simulink model "wlan_phy_rx_pmd"  (Simulink 7.8)
settings: Solver=VariableStepDiscrete  SolverMode=SingleTasking  StartTime=0.0  StopTime=rx_sim_time  RelTol=1e-3
PostLoadFcn:
  | wlan_phy_rx_init
InitFcn:
  | wlan_phy_rx_init
StartFcn:
  | wlan_phy_rx_init
values from neighbouring files (symbols this model uses):
  barker_seq20 = 0.95 * circshift(barker_seq20, [0 4]) ./ max(abs(barker_seq20))    [wlan_phy_rx_init.m]
  bit_scrambler_lfsr_bytes = bi2de(reshape(repmat(scr, 1, 8), 8, 127)', 'left-msb')    [wlan_phy_rx_init.m]
  deinterleave_ROM = [deinterleave_ROM interleave_64QAM zeros(1, 512-length(interleave_64QAM))]    [wlan_phy_rx_init.m]
  longCorr_coef_i = [3*real(longCorr_coef)]    [wlan_phy_rx_init.m]
  longCorr_coef_q = [3*imag(longCorr_coef)]    [wlan_phy_rx_init.m]
  MAX_CP_LEN = 32    [wlan_phy_rx_init.m]
  MAX_NCBPS = NCBPS_64QAM    (= 288)    [wlan_phy_rx_init.m]
  MAX_NUM_BYTES = 4096    [wlan_phy_rx_init.m]
  MAX_NUM_SC = 64    [wlan_phy_rx_init.m]
  MAX_NUM_SYMS = 600    [wlan_phy_rx_init.m]
  NCBPS_16QAM = 192    [wlan_phy_rx_init.m]
  NCBPS_64QAM = 288    [wlan_phy_rx_init.m]
  NCBPS_BPSK = 48    [wlan_phy_rx_init.m]
  NCBPS_QPSK = 96    [wlan_phy_rx_init.m]
  NDBPS_16QAM12 = 96    [wlan_phy_rx_init.m]
  NDBPS_16QAM34 = 144    [wlan_phy_rx_init.m]
  NDBPS_64QAM23 = 192    [wlan_phy_rx_init.m]
  NDBPS_64QAM34 = 216    [wlan_phy_rx_init.m]
  NDBPS_BPSK12 = 24    [wlan_phy_rx_init.m]
  NDBPS_BPSK34 = 36    [wlan_phy_rx_init.m]
  NDBPS_QPSK12 = 48    [wlan_phy_rx_init.m]
  NDBPS_QPSK34 = 72    [wlan_phy_rx_init.m]
  PHY_MIN_PKT_LEN = 14    [wlan_phy_rx_init.m]
  preFFT_sampBuff_numSamps = 4*MAX_NUM_SC    (= 256)    [wlan_phy_rx_init.m]
  rx_sim_sig_samp_time = 8    [wlan_phy_rx_init.m]
  scr_ind_rev = zeros(1,128)    [wlan_phy_rx_init.m]
undefined symbols (not defined in the file or in its folder): gcbh, xlBlockLoadCallback, xlGetNormalizedPeriod, xlipgetpartsetting
libraries: xbsIndex_r4 x3524, simulink x1, xbsEDKLib_r4 x1
(part 11 of 21)

== system wlan_phy_rx_pmd/Sync & Antenna Sel/LTS Correlation/Correlator ==
  note: The architecture for this correlator is based on one developed by Dr. Chris Dick (Cheif DSP Scientist at Xilinx).
      | The version here has been modified in a few ways:
      | -The inner correlators run at 8x the input sample rate (vs 5 in the original)
      | -The "multiplications" are 3-bit by 1-bit (vs 1x1 in the original), which improves
      | correlation performance with interference or overlapping preambles
  Rx I : Inport
  Rx Q : Inport  Port=2
  rx_IQ_valid : Inport  Port=3
  C1 : Subsystem  mask{N=8, h=longCorr_coef_i, Ts_In=8}
      mask prompts: N "Coefficients/correlator"; h "Coefficients"; Ts_In "Input sample period"
  C2 : Subsystem  mask{N=8, h=longCorr_coef_i, Ts_In=8}
      mask prompts: N "Coefficients/correlator"; h "Coefficients"; Ts_In "Input sample period"
  C3 : Subsystem  mask{N=8, h=longCorr_coef_q, Ts_In=8}
      mask prompts: N "Coefficients/correlator"; h "Coefficients"; Ts_In "Input sample period"
  C4 : Subsystem  mask{N=8, h=longCorr_coef_q, Ts_In=8}
      mask prompts: N "Coefficients/correlator"; h "Coefficients"; Ts_In "Input sample period"
  Combine : Subsystem
  Delay1 : xbsIndex_r4/Delay  rst=off  infoeditControl=Selection of Reset will increase slice count due to use of real FFs and instead of SRLs  en=on  latency=1  dbl_ovrd=off  reg_retiming=off  block_type=delay
  Delay2 : xbsIndex_r4/Delay  rst=off  infoeditControl=Selection of Reset will increase slice count due to use of real FFs and instead of SRLs  en=on  latency=1  dbl_ovrd=off  reg_retiming=off  block_type=delay
  Delay3 : xbsIndex_r4/Delay  rst=off  infoeditControl=Selection of Reset will increase slice count due to use of real FFs and instead of SRLs  en=off  latency=1  dbl_ovrd=off  reg_retiming=off  block_type=delay
  Delay4 : xbsIndex_r4/Delay  rst=off  infoeditControl=Selection of Reset will increase slice count due to use of real FFs and instead of SRLs  en=off  latency=1  dbl_ovrd=off  reg_retiming=off  block_type=delay
  FSM : Subsystem  mask{N=8}
      mask prompts: N "Coefficients/correlator"
  Goto Tag Visibility : GotoTagVisibility  GotoTag=ltsCorr_dAddr
  Goto Tag Visibility1 : GotoTagVisibility  GotoTag=ltsCorr_cAddr
  Goto Tag Visibility2 : GotoTagVisibility  GotoTag=ltsCorr_ld
  R : Outport
  tag scope: CS_LTS_Corr=global, ltsCorr_cAddr=scoped, ltsCorr_dAddr=scoped, ltsCorr_ld=scoped
  wires:
  rx_IQ_valid/1 -> Delay1/2, Delay2/2, Delay4/1, Delay3/1
  FSM/3(load) -> [ltsCorr_ld]
  FSM/2(Coef Addr) -> [ltsCorr_cAddr]
  FSM/1(Data Addr) -> [ltsCorr_dAddr]
  C3/1(y) -> Combine/2(Im*Im)
  Delay2/1 -> C2/1(x), C3/1(x)
  Delay1/1 -> C1/1(x), C4/1(x)
  C1/1(y) -> Combine/1(Re*Re)
  C2/1(y) -> Combine/4(Im*Re)
  C4/1(y) -> Combine/3(Re*Im)
  Combine/1(R) -> R/1, [CS_LTS_Corr]
  Rx I/1 -> Delay1/1
  Rx Q/1 -> Delay2/1
  Delay3/1 -> FSM/1(IQ_Valid)
  Delay4/1 -> C1/2(x_valid), C3/2(x_valid), C2/2(x_valid), C4/2(x_valid)

== system wlan_phy_rx_pmd/Sync & Antenna Sel/LTS Correlation/LTS Corr Timeout ==
  Pkt Det : Inport
  IQ Valid : Inport  Port=2
  LTS 1 : Inport  Port=3
  LTS 2 : Inport  Port=4
  Counter : xbsIndex_r4/Counter  cnt_type=Free Running  cnt_to=Inf  operation=Up  start_count=0  cnt_by_val=1  arith_type=Unsigned  n_bits=9  bin_pt=0  load_pin=off  rst=on  en=on  explicit_period=off  period=1  dbl_ovrd=off  use_behavioral_HDL=off  implementation=Fabric  block_type=counter
  Display : Display
  Gateway Out{1..9} (9 alike) : xbsIndex_r4/Gateway Out  inherit_from_input=off  hdl_port=off  timing_constraint=None  locs_specified=off  LOCs={}  UseAsDAC=off  DACChannel='1'  block_type=gatewayout
  Inverter : xbsIndex_r4/Inverter  en=off  latency=0  dbl_ovrd=off  block_type=inv
  LTS Timeout : Scope  NumInputPorts=10
      settings: TimeRange=18000  YMin=0~0~0~0~0~0~0~0~-1~-1  YMax=1~1~1~1~1~600~1~1~1~1  SaveName=ScopeData17
  Logical : xbsIndex_r4/Logical  logical_function=AND  inputs=2  en=off  latency=0  precision=Full  arith_type=Unsigned  n_bits=16  bin_pt=0  align_bp=on  dbl_ovrd=off  block_type=logical
  Logical1 : xbsIndex_r4/Logical  logical_function=NAND  inputs=2  en=off  latency=0  precision=Full  arith_type=Unsigned  n_bits=16  bin_pt=0  align_bp=on  dbl_ovrd=off  block_type=logical
  Logical2 : xbsIndex_r4/Logical  logical_function=AND  inputs=2  en=off  latency=0  precision=Full  arith_type=Unsigned  n_bits=16  bin_pt=0  align_bp=on  dbl_ovrd=off  block_type=logical
  Relational : xbsIndex_r4/Relational  mode=a>b  en=off  latency=0  dbl_ovrd=off  block_type=relational
  S-R Latch : Subsystem
  S-R Latch1 : Subsystem
  done14 : xbsIndex_r4/Gateway Out  inherit_from_input=off  hdl_port=off  timing_constraint=None  locs_specified=off  LOCs={}  UseAsDAC=off  DACChannel='1'  block_type=gatewayout
      settings: infoedit="Gateway out block.  Converts Xilinx fixed point inputs into ouputs of type Simulink integer, double, or fixed point.<P><P>Hardware notes:  In hardware these blocks become top level output ports or are discarded, depending on how they are configured."  xl_area=[0 0 0 0 1 0 0]  block_version=VER_STRING_GOES_HERE
  Timeout : Outport
  wires:
  Logical/1 -> Counter/2
  Pkt Det/1 -> Logical/1, Inverter/1, Gateway Out5/1
  IQ Valid/1 -> Logical/2
  Inverter/1 -> Counter/1, S-R Latch/2(R), S-R Latch1/2(R)
  LTS 1/1 -> S-R Latch/1(S), Gateway Out4/1
  LTS 2/1 -> S-R Latch1/1(S), Gateway Out1/1
  S-R Latch/1(Q) -> Logical1/1, Gateway Out2/1
  S-R Latch1/1(Q) -> Logical1/2, Gateway Out3/1
  Logical1/1 -> Logical2/1, Gateway Out8/1
  Relational/1 -> Logical2/2, Gateway Out9/1
  Logical2/1 -> Timeout/1, Gateway Out7/1
  Counter/1 -> Relational/1, Gateway Out6/1
  [regRx_PktDet_Timout] -> Relational/2, done14/1
  done14/1 -> Display/1
  Gateway Out4/1 -> LTS Timeout/1
  Gateway Out2/1 -> LTS Timeout/2
  Gateway Out1/1 -> LTS Timeout/3
  Gateway Out3/1 -> LTS Timeout/4
  Gateway Out5/1 -> LTS Timeout/5
  Gateway Out6/1 -> LTS Timeout/6
  Gateway Out7/1 -> LTS Timeout/10
  Gateway Out8/1 -> LTS Timeout/7
  Gateway Out9/1 -> LTS Timeout/8

== system wlan_phy_rx_pmd/Sync & Antenna Sel/LTS Correlation/Posedge3 ==
  note: Rising Edge Detection
  D : Inport
  Delay : xbsIndex_r4/Delay  rst=off  infoeditControl=Selection of Reset will increase slice count due to use of real FFs and instead of SRLs  en=off  latency=1  dbl_ovrd=off  reg_retiming=off  block_type=delay
      settings: infoedit=Hardware notes: A delay line is a chain, each link of which is an SRL16 followed by a flip-flop. If register retiming is enabled, the delay line is a chain of flip-flops.  xl_area=[1 1 0 0 0 0 0]  block_version=VER_STRING_GOES_HERE
  Inverter : xbsIndex_r4/Inverter  en=off  latency=0  dbl_ovrd=off  block_type=inv
      settings: xl_area=[0 0 0 0 0 0 0]  block_version=VER_STRING_GOES_HERE
  Logical1 : xbsIndex_r4/Logical  logical_function=AND  inputs=2  en=off  latency=0  precision=Full  arith_type=Unsigned  n_bits=8  bin_pt=2  align_bp=on  dbl_ovrd=off  block_type=logical
      settings: xl_area=[1 0 0 1 0 0 0]  block_version=VER_STRING_GOES_HERE
  Q : Outport
  wires:
  D/1 -> Logical1/2, Delay/1
  Logical1/1 -> Q/1
  Inverter/1 -> Logical1/1
  Delay/1 -> Inverter/1

== system wlan_phy_rx_pmd/Sync & Antenna Sel/LTS Correlation/S-R Latch ==
  S : Inport
  R : Inport  Port=2
  Constant1 : xbsIndex_r4/Constant  const=1  gui_display_data_type=Fixed-point  arith_type=Boolean  n_bits=1  bin_pt=0  preci_type=Single  exp_width=8  frac_width=24  explicit_period=off  period=1  equ=P=C  opselect=C  inp2=PCIN>>17  opr=+  inp1=P  carry=CIN  dbl_ovrd=off  block_type=constant
      settings: block_version=VER_STRING_GOES_HERE
  Convert1 : xbsIndex_r4/Convert  gui_display_data_type=Boolean  arith_type=Boolean  n_bits=1  bin_pt=0  float_type=Single  exp_bits=8  fraction_bits=24  quantization=Truncate  overflow=Wrap  en=off  latency=0  dbl_ovrd=off  pipeline=off  block_type=convert
  Convert2 : xbsIndex_r4/Convert  gui_display_data_type=Boolean  arith_type=Boolean  n_bits=1  bin_pt=0  float_type=Single  exp_bits=8  fraction_bits=24  quantization=Truncate  overflow=Wrap  en=off  latency=0  dbl_ovrd=off  pipeline=off  block_type=convert
  Register2 : xbsIndex_r4/Register  init=0  rst=on  en=on  dbl_ovrd=off  block_type=register
      settings: xl_area=[1 1 0 0 0 0 0]  block_version=VER_STRING_GOES_HERE
  Q : Outport
  wires:
  Convert2/1 -> Register2/3
  Convert1/1 -> Register2/2
  S/1 -> Convert2/1
  Register2/1 -> Q/1
  R/1 -> Convert1/1
  Constant1/1 -> Register2/1

== system wlan_phy_rx_pmd/Sync & Antenna Sel/LTS Correlation/S-R Latch1 ==
  S : Inport
  R : Inport  Port=2
  Constant1 : xbsIndex_r4/Constant  const=1  gui_display_data_type=Fixed-point  arith_type=Boolean  n_bits=1  bin_pt=0  preci_type=Single  exp_width=8  frac_width=24  explicit_period=off  period=1  equ=P=C  opselect=C  inp2=PCIN>>17  opr=+  inp1=P  carry=CIN  dbl_ovrd=off  block_type=constant
      settings: block_version=VER_STRING_GOES_HERE
  Convert1 : xbsIndex_r4/Convert  gui_display_data_type=Boolean  arith_type=Boolean  n_bits=1  bin_pt=0  float_type=Single  exp_bits=8  fraction_bits=24  quantization=Truncate  overflow=Wrap  en=off  latency=0  dbl_ovrd=off  pipeline=off  block_type=convert
  Convert2 : xbsIndex_r4/Convert  gui_display_data_type=Boolean  arith_type=Boolean  n_bits=1  bin_pt=0  float_type=Single  exp_bits=8  fraction_bits=24  quantization=Truncate  overflow=Wrap  en=off  latency=0  dbl_ovrd=off  pipeline=off  block_type=convert
  Register2 : xbsIndex_r4/Register  init=0  rst=on  en=on  dbl_ovrd=off  block_type=register
      settings: xl_area=[1 1 0 0 0 0 0]  block_version=VER_STRING_GOES_HERE
  Q : Outport
  wires:
  Constant1/1 -> Register2/1
  R/1 -> Convert1/1
  Register2/1 -> Q/1
  S/1 -> Convert2/1
  Convert1/1 -> Register2/2
  Convert2/1 -> Register2/3

== system wlan_phy_rx_pmd/Sync & Antenna Sel/LTS Correlation/Threshold Select ==
  Pkt Det : Inport
  RSSI : Inport  Port=2
  Mux : xbsIndex_r4/Mux  inputs=2  en=off  latency=0  precision=Full  arith_type=Unsigned  n_bits=16  bin_pt=14  quantization=Truncate  overflow=Wrap  dbl_ovrd=off  block_type=mux
  Register1 : xbsIndex_r4/Register  init=0  rst=off  en=on  dbl_ovrd=off  block_type=register
  Relational1 : xbsIndex_r4/Relational  mode=a>b  en=off  latency=0  dbl_ovrd=off  block_type=relational
      settings: xl_area=[10 0 0 19 0 0 0]  block_version=VER_STRING_GOES_HERE
  posedge : Subsystem
  Corr Thresh : Outport
  wires:
  Relational1/1 -> Mux/1
  RSSI/1 -> Register1/1
  posedge/1(Q) -> Register1/2
  Register1/1 -> Relational1/1
  [regRx_LongCorr_RSSI_Thresh] -> Relational1/2
  [regRx_LongCorr_Thresh_highSNR] -> Mux/3
  [regRx_LongCorr_Thresh_lowSNR] -> Mux/2
  Pkt Det/1 -> posedge/1(D)
  Mux/1 -> Corr Thresh/1

== system wlan_phy_rx_pmd/Sync & Antenna Sel/PHY CCA/PHY CCA Logic ==
  note: Two modes for physical carrier sensing:
      | Mode 0: Any enabled antenna can assert BUSY if its RSSI exceeds the threshold
      | Mode 1: BUSY only if *all* enabled antennas exceed the threshold
  note: This 4-in OR deasserts BUSY
      | if all antennas are disabled.
  Det A : Inport
  Det B : Inport  Port=2
  Det C : Inport  Port=3
  Det D : Inport  Port=4
  Inverter{,1..3} (4 alike) : xbsIndex_r4/Inverter  en=off  latency=0  dbl_ovrd=off  block_type=inv
  Logical{,2..4} (4 alike) : xbsIndex_r4/Logical  logical_function=AND  inputs=2  en=off  latency=0  precision=Full  arith_type=Unsigned  n_bits=16  bin_pt=0  align_bp=on  dbl_ovrd=off  block_type=logical
  Logical1 : xbsIndex_r4/Logical  logical_function=OR  inputs=4  en=off  latency=0  precision=Full  arith_type=Unsigned  n_bits=16  bin_pt=0  align_bp=on  dbl_ovrd=off  block_type=logical
  Logical10 : xbsIndex_r4/Logical  logical_function=OR  inputs=4  en=off  latency=0  precision=Full  arith_type=Unsigned  n_bits=16  bin_pt=0  align_bp=on  dbl_ovrd=off  block_type=logical
  Logical5 : xbsIndex_r4/Logical  logical_function=AND  inputs=5  en=off  latency=0  precision=Full  arith_type=Unsigned  n_bits=16  bin_pt=0  align_bp=on  dbl_ovrd=off  block_type=logical
  Logical{6..9} (4 alike) : xbsIndex_r4/Logical  logical_function=OR  inputs=2  en=off  latency=0  precision=Full  arith_type=Unsigned  n_bits=16  bin_pt=0  align_bp=on  dbl_ovrd=off  block_type=logical
  Mux : xbsIndex_r4/Mux  inputs=2  en=off  latency=0  precision=Full  arith_type=Unsigned  n_bits=16  bin_pt=14  quantization=Truncate  overflow=Wrap  dbl_ovrd=off  block_type=mux
  BUSY : Outport
  wires:
  [regRx_pktDetEn_RFA] -> Logical/1
  Logical/1 -> Logical1/1, Logical6/1
  Logical2/1 -> Logical1/2, Logical7/1
  Logical1/1 -> Mux/2
  Det A/1 -> Logical/2
  Det B/1 -> Logical2/2
  [regRx_pktDetEn_RFB] -> Logical2/1
  Det C/1 -> Logical3/2
  Det D/1 -> Logical4/2
  [regRx_pktDetEn_RFC] -> Logical3/1
  [regRx_pktDetEn_RFD] -> Logical4/1
  Logical3/1 -> Logical1/3, Logical8/1
  Logical4/1 -> Logical1/4, Logical9/1
  Inverter/1 -> Logical6/2
  [regRx_pktDetEn_RFA] -> Inverter/1, Logical10/1
  Logical6/1 -> Logical5/1
  Logical7/1 -> Logical5/2
  [regRx_pktDetEn_RFB] -> Inverter1/1, Logical10/2
  Inverter1/1 -> Logical7/2
  Logical8/1 -> Logical5/3
  [regRx_pktDetEn_RFC] -> Inverter2/1, Logical10/3
  Inverter2/1 -> Logical8/2
  [regRx_pktDetEn_RFD] -> Inverter3/1, Logical10/4
  Inverter3/1 -> Logical9/2
  Logical9/1 -> Logical5/4
  Logical10/1 -> Logical5/5
  Logical5/1 -> Mux/3
  Mux/1 -> BUSY/1
  [regRx_phyCCA_Mode_Sel] -> Mux/1

== system wlan_phy_rx_pmd/Sync & Antenna Sel/PHY CCA/Rate*Length BUSY ==
  note: CCA_BUSY for any Rx pkt with valid SIGNAL field
      | Rx Active drops after last data-bearing sample plus
      | programmable extension. This block doesn't care about
      | actual decoding latency or supported rates.
      |
      | This block starts counting before the SIGNAL field is
      | decoded. If the SIGNAL field is invalid, the default rate/length
      | (24 bits @ 6Mbps) will be used. This will de-assert busy after
      | the symbols containing the undecodable SIGNAL field.
  IQ Valid : Inport
  Start : Inport  Port=2
  Bits : Subsystem
  Extension : Subsystem
  Gateway Out{1..2,4..5,8..9} (6 alike) : xbsIndex_r4/Gateway Out  inherit_from_input=off  hdl_port=off  timing_constraint=None  locs_specified=off  LOCs={}  UseAsDAC=off  DACChannel='1'  block_type=gatewayout
  OFDM Syms : Subsystem
  PHY Fake Active : Scope  NumInputPorts=7
      settings: TimeRange=25000  YMin=0~0~0~-1~0~0~0  YMax=1~1~1~1~1~1~1  SaveName=ScopeData30
  S-R Latch2 : Subsystem
  PHY Rx Active : Outport
  tag scope: RX_END_OFDM_LAST_SAMP=global
  wires:
  Gateway Out4/1 -> PHY Fake Active/1
  Gateway Out1/1 -> PHY Fake Active/2
  Gateway Out2/1 -> PHY Fake Active/3
  IQ Valid/1 -> OFDM Syms/2(IQ valid), Extension/2(IQ Valid)
  Gateway Out5/1 -> PHY Fake Active/5
  Gateway Out8/1 -> PHY Fake Active/6
  Gateway Out9/1 -> PHY Fake Active/7
  Start/1 -> Gateway Out4/1, Extension/3(Rst), Bits/2(Rst), OFDM Syms/1(Start)
  S-R Latch2/1(Q) -> PHY Rx Active/1, Gateway Out9/1
  OFDM Syms/1(Sym) -> Bits/1(Sym Inc), Gateway Out1/1
  [RX_START_OFDM_SIGNAL_VALID] -> S-R Latch2/1(S), Gateway Out5/1
  Extension/1(Pkt Done) -> S-R Latch2/2(R), Gateway Out8/1, [RX_END_OFDM_LAST_SAMP], OFDM Syms/3(Rst)
  Bits/1(Done) -> Extension/1(Bits Done), Gateway Out2/1

== system wlan_phy_rx_pmd/Sync & Antenna Sel/PHY CCA/S-R Latch2 ==
  S : Inport
  R : Inport  Port=2
  Constant1 : xbsIndex_r4/Constant  const=1  gui_display_data_type=Fixed-point  arith_type=Boolean  n_bits=1  bin_pt=0  preci_type=Single  exp_width=8  frac_width=24  explicit_period=off  period=1  equ=P=C  opselect=C  inp2=PCIN>>17  opr=+  inp1=P  carry=CIN  dbl_ovrd=off  block_type=constant
      settings: block_version=VER_STRING_GOES_HERE
  Convert1 : xbsIndex_r4/Convert  gui_display_data_type=Boolean  arith_type=Boolean  n_bits=1  bin_pt=0  float_type=Single  exp_bits=8  fraction_bits=24  quantization=Truncate  overflow=Wrap  en=off  latency=0  dbl_ovrd=off  pipeline=off  block_type=convert
  Convert2 : xbsIndex_r4/Convert  gui_display_data_type=Boolean  arith_type=Boolean  n_bits=1  bin_pt=0  float_type=Single  exp_bits=8  fraction_bits=24  quantization=Truncate  overflow=Wrap  en=off  latency=0  dbl_ovrd=off  pipeline=off  block_type=convert
  Register2 : xbsIndex_r4/Register  init=0  rst=on  en=on  dbl_ovrd=off  block_type=register
      settings: xl_area=[1 1 0 0 0 0 0]  block_version=VER_STRING_GOES_HERE
  Q : Outport
  wires:
  Convert2/1 -> Register2/3
  Convert1/1 -> Register2/2
  S/1 -> Convert2/1
  Register2/1 -> Q/1
  R/1 -> Convert1/1
  Constant1/1 -> Register2/1

== system wlan_phy_rx_pmd/Sync & Antenna Sel/Pkt Det/DSSS Ant Sel Logic ==
  Det A : Inport
  Det B : Inport  Port=2
  Det C : Inport  Port=3
  Det D : Inport  Port=4
  Concat : xbsIndex_r4/Concat  num_inputs=4  dbl_ovrd=off  block_type=concat
  Logical{,2..4} (4 alike) : xbsIndex_r4/Logical  logical_function=AND  inputs=2  en=off  latency=0  precision=Full  arith_type=Unsigned  n_bits=16  bin_pt=0  align_bp=on  dbl_ovrd=off  block_type=logical
  Logical1 : xbsIndex_r4/Logical  logical_function=OR  inputs=4  en=off  latency=0  precision=Full  arith_type=Unsigned  n_bits=16  bin_pt=0  align_bp=on  dbl_ovrd=off  block_type=logical
  Pkt Det : Outport
  tag scope: PKTDET_STATUS_DSSS=global
  wires:
  Logical4/1 -> Logical1/4, Concat/4
  Logical3/1 -> Logical1/3, Concat/3
  Det D/1 -> Logical4/2
  Det C/1 -> Logical3/2
  [regRx_pktDetEn_RFD] -> Logical4/1
  [regRx_pktDetEn_RFC] -> Logical3/1
  [regRx_pktDetEn_RFB] -> Logical2/1
  Det B/1 -> Logical2/2
  Det A/1 -> Logical/2
  Logical1/1 -> Pkt Det/1
  Logical2/1 -> Logical1/2, Concat/2
  Logical/1 -> Logical1/1, Concat/1
  [regRx_pktDetEn_RFA] -> Logical/1
  Concat/1 -> [PKTDET_STATUS_DSSS]

== system wlan_phy_rx_pmd/Sync & Antenna Sel/Pkt Det/Ext Pkt Det ==
  Reset : Inport
  Constant : Constant  Value=0
  Convert : xbsIndex_r4/Convert  gui_display_data_type=Boolean  arith_type=Boolean  n_bits=16  bin_pt=14  float_type=Single  exp_bits=8  fraction_bits=24  quantization=Truncate  overflow=Wrap  en=off  latency=0  dbl_ovrd=off  pipeline=off  block_type=convert
  Convert1 : xbsIndex_r4/Convert  gui_display_data_type=Boolean  arith_type=Boolean  n_bits=16  bin_pt=14  float_type=Single  exp_bits=8  fraction_bits=24  quantization=Truncate  overflow=Wrap  en=off  latency=0  dbl_ovrd=off  pipeline=off  block_type=convert
  Convert2 : xbsIndex_r4/Convert  gui_display_data_type=Boolean  arith_type=Boolean  n_bits=16  bin_pt=14  float_type=Single  exp_bits=8  fraction_bits=24  quantization=Truncate  overflow=Wrap  en=off  latency=0  dbl_ovrd=off  pipeline=off  block_type=convert
  Debouce : Subsystem
  Logical1 : xbsIndex_r4/Logical  logical_function=AND  inputs=2  en=off  latency=0  precision=Full  arith_type=Unsigned  n_bits=16  bin_pt=0  align_bp=on  dbl_ovrd=off  block_type=logical
  Posedge1 : Subsystem
  Register{1..5} (5 alike) : xbsIndex_r4/Register  init=0  rst=off  en=off  dbl_ovrd=off  block_type=register
  S-R Latch : Subsystem
  pkt_det_in : xbsIndex_r4/Gateway In  gui_display_data_type=Fixed-point  arith_type=Unsigned  n_bits=1  bin_pt=0  preci_type=Single  exp_width=8  frac_width=24  quantization="Round  (unbiased: +/- Inf)"  overflow=Saturate  period=1  dbl_ovrd=off  timing_constraint=None  locs_specified=off  LOCs={}  UseAsADC=off  ADCChannel='1'  inherit_from_input=off  hdl_port=on  block_type=gatewayin
  Det : Outport
  wires:
  Constant/1 -> pkt_det_in/1
  S-R Latch/1(Q) -> Logical1/2
  Posedge1/1(Q) -> Convert1/1
  Reset/1 -> Register5/1
  Debouce/1(Q) -> Posedge1/1(D)
  pkt_det_in/1 -> Register4/1
  Logical1/1 -> Det/1
  [regRx_pktDetEn_Ext] -> Convert2/1
  Convert/1 -> S-R Latch/1(R)
  Convert1/1 -> S-R Latch/2(S)
  Convert2/1 -> Logical1/1
  Register4/1 -> Register1/1
  Register1/1 -> Register2/1
  Register2/1 -> Debouce/1(D)
  Register3/1 -> Debouce/2(R), Convert/1
  Register5/1 -> Register3/1

== system wlan_phy_rx_pmd/Sync & Antenna Sel/Pkt Det/OFDM Ant Sel Logic ==
  Ext Det : Inport
  Det A : Inport  Port=2
  Det B : Inport  Port=3
  Det C : Inport  Port=4
  Det D : Inport  Port=5
  Concat : xbsIndex_r4/Concat  num_inputs=5  dbl_ovrd=off  block_type=concat
  Logical{,2..4} (4 alike) : xbsIndex_r4/Logical  logical_function=AND  inputs=2  en=off  latency=0  precision=Full  arith_type=Unsigned  n_bits=16  bin_pt=0  align_bp=on  dbl_ovrd=off  block_type=logical
  Logical1 : xbsIndex_r4/Logical  logical_function=OR  inputs=4  en=off  latency=0  precision=Full  arith_type=Unsigned  n_bits=16  bin_pt=0  align_bp=on  dbl_ovrd=off  block_type=logical
  Pkt Det : Outport
  tag scope: PKTDET_STATUS_OFDM=global
  wires:
  [regRx_pktDetEn_RFA] -> Logical/1
  Logical/1 -> Logical1/1, Concat/5
  Logical2/1 -> Logical1/2, Concat/4
  Logical1/1 -> Pkt Det/1
  Det A/1 -> Logical/2
  Det B/1 -> Logical2/2
  [regRx_pktDetEn_RFB] -> Logical2/1
  [regRx_pktDetEn_RFC] -> Logical3/1
  [regRx_pktDetEn_RFD] -> Logical4/1
  Det C/1 -> Logical3/2
  Det D/1 -> Logical4/2
  Logical3/1 -> Logical1/3, Concat/3
  Logical4/1 -> Logical1/4, Concat/2
  Concat/1 -> [PKTDET_STATUS_OFDM]
  Ext Det/1 -> Concat/1

== system wlan_phy_rx_pmd/Sync & Antenna Sel/Pkt Det/RF A Det ==
  Reset OFDM : Inport
  Reset DSSS : Inport  Port=2
  Rx I : Inport  Port=3
  Rx Q : Inport  Port=4
  IQ Valid : Inport  Port=5
  RSSI : Inport  Port=6
  Auto-Corr Pkt Det : Subsystem
  Logical : xbsIndex_r4/Logical  logical_function=OR  inputs=2  en=off  latency=0  precision=Full  arith_type=Unsigned  n_bits=16  bin_pt=0  align_bp=on  dbl_ovrd=off  block_type=logical
  Logical1 : xbsIndex_r4/Logical  logical_function=OR  inputs=2  en=off  latency=0  precision=Full  arith_type=Unsigned  n_bits=16  bin_pt=0  align_bp=on  dbl_ovrd=off  block_type=logical
  Logical2 : xbsIndex_r4/Logical  logical_function=AND  inputs=2  en=off  latency=0  precision=Full  arith_type=Unsigned  n_bits=16  bin_pt=0  align_bp=on  dbl_ovrd=off  block_type=logical
  Logical3 : xbsIndex_r4/Logical  logical_function=AND  inputs=2  en=off  latency=0  precision=Full  arith_type=Unsigned  n_bits=16  bin_pt=0  align_bp=on  dbl_ovrd=off  block_type=logical
  Mux : xbsIndex_r4/Mux  inputs=2  en=off  latency=0  precision=Full  arith_type=Unsigned  n_bits=16  bin_pt=14  quantization=Truncate  overflow=Wrap  dbl_ovrd=off  block_type=mux
  Mux1 : xbsIndex_r4/Mux  inputs=2  en=off  latency=0  precision=Full  arith_type=Unsigned  n_bits=16  bin_pt=14  quantization=Truncate  overflow=Wrap  dbl_ovrd=off  block_type=mux
  RSSI Pkt Det : Subsystem
  Det OFDM : Outport
  Det DSSS : Outport  Port=2
  wires:
  Logical/1 -> Mux/2
  IQ Valid/1 -> Auto-Corr Pkt Det/5(rx_valid)
  Rx Q/1 -> Auto-Corr Pkt Det/4(rx_Q)
  Rx I/1 -> Auto-Corr Pkt Det/3(rx_I)
  Logical1/1 -> Mux1/2
  Reset OFDM/1 -> Auto-Corr Pkt Det/1(Reset OFDM), RSSI Pkt Det/1(Reset OFDM)
  Reset DSSS/1 -> Auto-Corr Pkt Det/2(Reset DSSS), RSSI Pkt Det/2(Reset DSSS)
  [regRx_pktDet_requireBothA] -> Mux/1, Mux1/1
  Logical3/1 -> Mux/3
  Mux/1 -> Det OFDM/1
  RSSI/1 -> RSSI Pkt Det/3(Avg RSSI)
  Auto-Corr Pkt Det/2(Det DSSS) -> Logical1/1, Logical2/1
  RSSI Pkt Det/2(PktDet DSSS) -> Logical1/2, Logical2/2
  Auto-Corr Pkt Det/1(Det OFDM) -> Logical/1, Logical3/1
  RSSI Pkt Det/1(PktDet OFDM) -> Logical/2, Logical3/2
  Logical2/1 -> Mux1/3
  Mux1/1 -> Det DSSS/1

== system wlan_phy_rx_pmd/Sync & Antenna Sel/Pkt Det/RF B Det ==
  Reset OFDM : Inport
  Reset DSSS : Inport  Port=2
  Rx I : Inport  Port=3
  Rx Q : Inport  Port=4
  IQ Valid : Inport  Port=5
  RSSI : Inport  Port=6
  Auto-Corr Pkt Det : Subsystem
  Logical : xbsIndex_r4/Logical  logical_function=OR  inputs=2  en=off  latency=0  precision=Full  arith_type=Unsigned  n_bits=16  bin_pt=0  align_bp=on  dbl_ovrd=off  block_type=logical
  Logical1 : xbsIndex_r4/Logical  logical_function=OR  inputs=2  en=off  latency=0  precision=Full  arith_type=Unsigned  n_bits=16  bin_pt=0  align_bp=on  dbl_ovrd=off  block_type=logical
  Logical2 : xbsIndex_r4/Logical  logical_function=AND  inputs=2  en=off  latency=0  precision=Full  arith_type=Unsigned  n_bits=16  bin_pt=0  align_bp=on  dbl_ovrd=off  block_type=logical
  Logical3 : xbsIndex_r4/Logical  logical_function=AND  inputs=2  en=off  latency=0  precision=Full  arith_type=Unsigned  n_bits=16  bin_pt=0  align_bp=on  dbl_ovrd=off  block_type=logical
  Mux : xbsIndex_r4/Mux  inputs=2  en=off  latency=0  precision=Full  arith_type=Unsigned  n_bits=16  bin_pt=14  quantization=Truncate  overflow=Wrap  dbl_ovrd=off  block_type=mux
  Mux1 : xbsIndex_r4/Mux  inputs=2  en=off  latency=0  precision=Full  arith_type=Unsigned  n_bits=16  bin_pt=14  quantization=Truncate  overflow=Wrap  dbl_ovrd=off  block_type=mux
  RSSI Pkt Det : Subsystem
  Det OFDM : Outport
  Det DSSS : Outport  Port=2
  wires:
  RSSI Pkt Det/1(PktDet OFDM) -> Logical3/2, Logical/2
  Auto-Corr Pkt Det/1(Det OFDM) -> Logical3/1, Logical/1
  RSSI Pkt Det/2(PktDet DSSS) -> Logical1/2, Logical2/2
  Auto-Corr Pkt Det/2(Det DSSS) -> Logical1/1, Logical2/1
  RSSI/1 -> RSSI Pkt Det/3(Avg RSSI)
  Mux/1 -> Det OFDM/1
  Logical3/1 -> Mux/3
  [regRx_pktDet_requireBothB] -> Mux/1, Mux1/1
  Reset DSSS/1 -> RSSI Pkt Det/2(Reset DSSS), Auto-Corr Pkt Det/2(Reset DSSS)
  Reset OFDM/1 -> RSSI Pkt Det/1(Reset OFDM), Auto-Corr Pkt Det/1(Reset OFDM)
  Rx I/1 -> Auto-Corr Pkt Det/3(rx_I)
  Rx Q/1 -> Auto-Corr Pkt Det/4(rx_Q)
  IQ Valid/1 -> Auto-Corr Pkt Det/5(rx_valid)
  Logical/1 -> Mux/2
  Mux1/1 -> Det DSSS/1
  Logical1/1 -> Mux1/2
  Logical2/1 -> Mux1/3

== system wlan_phy_rx_pmd/Sync & Antenna Sel/Resets/Reset Gen ==
  note: Extend pkt det reset by programmable
      | duration, to avoid false positives after
      | actual receptions due to Rx transients
      | during AGC reset
  Done : Inport
  Concat : xbsIndex_r4/Concat  num_inputs=2  dbl_ovrd=off  block_type=concat
  Constant : xbsIndex_r4/Constant  const=0  gui_display_data_type=Fixed-point  arith_type=Unsigned  n_bits=4  bin_pt=0  preci_type=Single  exp_width=8  frac_width=24  explicit_period=off  period=1  equ=P=C  opselect=C  inp2=PCIN>>17  opr=+  inp1=P  carry=CIN  dbl_ovrd=off  block_type=constant
  Counter1 : xbsIndex_r4/Counter  cnt_type=Free Running  cnt_to=Inf  operation=Up  start_count=0  cnt_by_val=1  arith_type=Unsigned  n_bits=10  bin_pt=0  load_pin=off  rst=on  en=on  explicit_period=off  period=4  dbl_ovrd=off  use_behavioral_HDL=off  implementation=Fabric  use_rpm=off  block_type=counter
  Inverter : xbsIndex_r4/Inverter  en=off  latency=0  dbl_ovrd=off  block_type=inv
      settings: xl_area=[0 0 0 0 0 0 0]  block_version=VER_STRING_GOES_HERE
  Logical2 : xbsIndex_r4/Logical  logical_function=OR  inputs=3  en=off  latency=0  precision=Full  arith_type=Unsigned  n_bits=16  bin_pt=0  align_bp=on  dbl_ovrd=off  block_type=logical
  Register : xbsIndex_r4/Register  init=0  rst=off  en=off  dbl_ovrd=off  block_type=register
  Relational3 : xbsIndex_r4/Relational  mode=a>b  en=off  latency=0  dbl_ovrd=off  block_type=relational
      settings: block_version=VER_STRING_GOES_HERE
  S-R Latch2 : Subsystem
  negedge : Subsystem
  Reset : Outport
  wires:
  Done/1 -> Logical2/2, negedge/1(D)
  negedge/1(Q) -> S-R Latch2/1(S), Logical2/3
  S-R Latch2/1(Q) -> Counter1/2, Logical2/1, Inverter/1
  Counter1/1 -> Relational3/1
  Relational3/1 -> S-R Latch2/2(R)
  Logical2/1 -> Reset/1
  Concat/1 -> Relational3/2
  Constant/1 -> Concat/2
  [regRx_PKT_DET_RESET_EXT_DUR] -> Register/1
  Register/1 -> Concat/1
  Inverter/1 -> Counter1/1

== system wlan_phy_rx_pmd/Sync & Antenna Sel/Resets/Reset Gen1 ==
  note: Extend pkt det reset by programmable
      | duration, to avoid false positives after
      | actual receptions due to Rx transients
      | during AGC reset
  Done : Inport
  Concat : xbsIndex_r4/Concat  num_inputs=2  dbl_ovrd=off  block_type=concat
  Constant : xbsIndex_r4/Constant  const=0  gui_display_data_type=Fixed-point  arith_type=Unsigned  n_bits=4  bin_pt=0  preci_type=Single  exp_width=8  frac_width=24  explicit_period=off  period=1  equ=P=C  opselect=C  inp2=PCIN>>17  opr=+  inp1=P  carry=CIN  dbl_ovrd=off  block_type=constant
  Counter1 : xbsIndex_r4/Counter  cnt_type=Free Running  cnt_to=Inf  operation=Up  start_count=0  cnt_by_val=1  arith_type=Unsigned  n_bits=10  bin_pt=0  load_pin=off  rst=on  en=on  explicit_period=off  period=4  dbl_ovrd=off  use_behavioral_HDL=off  implementation=Fabric  use_rpm=off  block_type=counter
  Inverter : xbsIndex_r4/Inverter  en=off  latency=0  dbl_ovrd=off  block_type=inv
      settings: xl_area=[0 0 0 0 0 0 0]  block_version=VER_STRING_GOES_HERE
  Logical2 : xbsIndex_r4/Logical  logical_function=OR  inputs=3  en=off  latency=0  precision=Full  arith_type=Unsigned  n_bits=16  bin_pt=0  align_bp=on  dbl_ovrd=off  block_type=logical
  Register : xbsIndex_r4/Register  init=0  rst=off  en=off  dbl_ovrd=off  block_type=register
  Relational3 : xbsIndex_r4/Relational  mode=a>b  en=off  latency=0  dbl_ovrd=off  block_type=relational
      settings: block_version=VER_STRING_GOES_HERE
  S-R Latch2 : Subsystem
  negedge : Subsystem
  Reset : Outport
  wires:
  Inverter/1 -> Counter1/1
  Register/1 -> Concat/1
  [regRx_PKT_DET_RESET_EXT_DUR] -> Register/1
  Constant/1 -> Concat/2
  Concat/1 -> Relational3/2
  Logical2/1 -> Reset/1
  Relational3/1 -> S-R Latch2/2(R)
  Counter1/1 -> Relational3/1
  S-R Latch2/1(Q) -> Inverter/1, Logical2/1, Counter1/2
  negedge/1(Q) -> Logical2/3, S-R Latch2/1(S)
  Done/1 -> negedge/1(D), Logical2/2

== system wlan_phy_rx_pmd/CFO & Samp Buffer/CFO Correction/Sim Reset/Subsystem ==
  Disregard Subsystem : xbsIndex_r4/Disregard Subsystem  Tag=discardX  block_type=disregard
  Gateway In : xbsIndex_r4/Gateway In  gui_display_data_type=Boolean  arith_type=Boolean  n_bits=16  bin_pt=14  preci_type=Single  exp_width=8  frac_width=24  quantization="Round  (unbiased: +/- Inf)"  overflow=Saturate  period=1  dbl_ovrd=off  timing_constraint=None  locs_specified=off  LOCs={}  UseAsADC=off  ADCChannel='1'  inherit_from_input=off  hdl_port=on  block_type=gatewayin
  Pulse Generator : DiscretePulseGenerator  Period=1e9  PulseWidth=16  PhaseDelay=1
  Out1 : Outport
  wires:
  Pulse Generator/1 -> Gateway In/1
  Gateway In/1 -> Out1/1

== system wlan_phy_rx_pmd/CFO & Samp Buffer/Samp Buffer/FFT Load Ctrl/Count-To Calc ==
  Skip Sel : Inport
  AddSub1 : xbsIndex_r4/AddSub  mode=Addition  use_carryin=off  use_carryout=off  en=off  latency=0  precision=User Defined  arith_type=Unsigned  n_bits=ceil(log2(preFFT_sampBuff_numSamps))  bin_pt=0  quantization=Truncate  overflow=Wrap  dbl_ovrd=off  use_behavioral_HDL=off  hw_selection=Fabric  pipelined=off  block_type=addsub
  Constant4 : xbsIndex_r4/Constant  const=0  gui_display_data_type=Fixed-point  arith_type=Unsigned  n_bits=1  bin_pt=0  preci_type=Single  exp_width=8  frac_width=24  explicit_period=off  period=8  equ=P=C  opselect=C  inp2=PCIN>>17  opr=+  inp1=P  carry=CIN  dbl_ovrd=off  block_type=constant
  Display : Display
  Display1 : Display
  Gateway Out : xbsIndex_r4/Gateway Out  inherit_from_input=off  hdl_port=off  timing_constraint=None  locs_specified=off  LOCs={}  UseAsDAC=off  DACChannel='1'  block_type=gatewayout
  Gateway Out1 : xbsIndex_r4/Gateway Out  inherit_from_input=off  hdl_port=off  timing_constraint=None  locs_specified=off  LOCs={}  UseAsDAC=off  DACChannel='1'  block_type=gatewayout
  Mux : xbsIndex_r4/Mux  inputs=2  en=off  latency=0  precision=Full  arith_type=Unsigned  n_bits=16  bin_pt=14  quantization=Truncate  overflow=Wrap  dbl_ovrd=off  block_type=mux
  Samp+Skip : Outport
  Samp : Outport  Port=2
  wires:
  [regRx_CP_LEN] -> Mux/3, Gateway Out/1
  Skip Sel/1 -> Mux/1
  Constant4/1 -> Mux/2
  Mux/1 -> AddSub1/1
  AddSub1/1 -> Samp+Skip/1
  [regRx_NUM_SC] -> AddSub1/2, Samp/1, Gateway Out1/1
  Gateway Out/1 -> Display/1
  Gateway Out1/1 -> Display1/1

== system wlan_phy_rx_pmd/CFO & Samp Buffer/Samp Buffer/FFT Load Ctrl/First Samp Calc ==
  Wr Addr : Inport
  LTS Sync : Inport  Port=2
  AddSub2 : xbsIndex_r4/AddSub  mode=Subtraction  use_carryin=off  use_carryout=off  en=off  latency=0  precision=User Defined  arith_type=Unsigned  n_bits=ceil(log2(preFFT_sampBuff_numSamps))  bin_pt=0  quantization=Truncate  overflow=Wrap  dbl_ovrd=off  use_behavioral_HDL=off  hw_selection=Fabric  pipelined=off  block_type=addsub
  AddSub3 : xbsIndex_r4/AddSub  mode=Addition  use_carryin=off  use_carryout=off  en=off  latency=0  precision=User Defined  arith_type=Unsigned  n_bits=ceil(log2(preFFT_sampBuff_numSamps))  bin_pt=0  quantization=Truncate  overflow=Wrap  dbl_ovrd=off  use_behavioral_HDL=off  hw_selection=Fabric  pipelined=off  block_type=addsub
  Register : xbsIndex_r4/Register  init=0  rst=off  en=on  dbl_ovrd=off  block_type=register
  Shift : xbsIndex_r4/Shift  shift_dir=Left  shift_bits=1  en=off  latency=0  precision=Full  arith_type="Signed  (2's comp)"  n_bits=16  bin_pt=14  quantization=Truncate  overflow=Wrap  dbl_ovrd=off  block_type=shift
  Samp Addr : Outport
  wires:
  [regRx_NUM_SC] -> Shift/1
  Shift/1 -> AddSub3/1
  AddSub3/1 -> AddSub2/2
  Register/1 -> AddSub2/1
  Wr Addr/1 -> Register/1
  AddSub2/1 -> Samp Addr/1
  LTS Sync/1 -> Register/2
  [regRx_FFT_OFFSET] -> AddSub3/2

== system wlan_phy_rx_pmd/CFO & Samp Buffer/Samp Buffer/FFT Load Ctrl/Posedge ==
  note: Rising Edge Detection
  D : Inport
  Delay : xbsIndex_r4/Delay  rst=off  infoeditControl=Selection of Reset will increase slice count due to use of real FFs and instead of SRLs  en=off  latency=1  dbl_ovrd=off  reg_retiming=off  block_type=delay
      settings: infoedit=Hardware notes: A delay line is a chain, each link of which is an SRL16 followed by a flip-flop. If register retiming is enabled, the delay line is a chain of flip-flops.  xl_area=[1 1 0 0 0 0 0]  block_version=VER_STRING_GOES_HERE
  Inverter : xbsIndex_r4/Inverter  en=off  latency=0  dbl_ovrd=off  block_type=inv
      settings: xl_area=[0 0 0 0 0 0 0]  block_version=VER_STRING_GOES_HERE
  Logical1 : xbsIndex_r4/Logical  logical_function=AND  inputs=2  en=off  latency=0  precision=Full  arith_type=Unsigned  n_bits=8  bin_pt=2  align_bp=on  dbl_ovrd=off  block_type=logical
      settings: xl_area=[1 0 0 1 0 0 0]  block_version=VER_STRING_GOES_HERE
  Q : Outport
  wires:
  D/1 -> Logical1/2, Delay/1
  Logical1/1 -> Q/1
  Inverter/1 -> Logical1/1
  Delay/1 -> Inverter/1

== system wlan_phy_rx_pmd/CFO & Samp Buffer/Samp Buffer/FFT Load Ctrl/S-R Latch1 ==
  S : Inport
  R : Inport  Port=2
  Constant1 : xbsIndex_r4/Constant  const=1  gui_display_data_type=Fixed-point  arith_type=Boolean  n_bits=1  bin_pt=0  preci_type=Single  exp_width=8  frac_width=24  explicit_period=off  period=1  equ=P=C  opselect=C  inp2=PCIN>>17  opr=+  inp1=P  carry=CIN  dbl_ovrd=off  block_type=constant
      settings: block_version=VER_STRING_GOES_HERE
  Convert1 : xbsIndex_r4/Convert  gui_display_data_type=Boolean  arith_type=Boolean  n_bits=1  bin_pt=0  float_type=Single  exp_bits=8  fraction_bits=24  quantization=Truncate  overflow=Wrap  en=off  latency=0  dbl_ovrd=off  pipeline=off  block_type=convert
  Convert2 : xbsIndex_r4/Convert  gui_display_data_type=Boolean  arith_type=Boolean  n_bits=1  bin_pt=0  float_type=Single  exp_bits=8  fraction_bits=24  quantization=Truncate  overflow=Wrap  en=off  latency=0  dbl_ovrd=off  pipeline=off  block_type=convert
  Register2 : xbsIndex_r4/Register  init=0  rst=on  en=on  dbl_ovrd=off  block_type=register
      settings: xl_area=[1 1 0 0 0 0 0]  block_version=VER_STRING_GOES_HERE
  Q : Outport
  wires:
  S/1 -> Convert2/1
  Register2/1 -> Q/1
  R/1 -> Convert1/1
  Constant1/1 -> Register2/1
  Convert1/1 -> Register2/2
  Convert2/1 -> Register2/3

== system wlan_phy_rx_pmd/Chan Est & EQ/EQ & Pilot Processing/Debug Outputs/4x Buffer ==
  I : Inport
  Q : Inport  Port=2
  Vin : Inport  Port=3
  14LSB : xbsIndex_r4/Slice  nbits=14  boolean_output=off  mode=Lower Bit Location + Width  bit1=0  base1=MSB of Input  bit0=0  base0=LSB of Input  dbl_ovrd=off  block_type=slice
  14MSB : xbsIndex_r4/Slice  nbits=14  boolean_output=off  mode=Upper Bit Location + Width  bit1=0  base1=MSB of Input  bit0=0  base0=LSB of Input  dbl_ovrd=off  block_type=slice
  Concat : xbsIndex_r4/Concat  num_inputs=2  dbl_ovrd=off  block_type=concat
  Constant3 : xbsIndex_r4/Constant  const=1  gui_display_data_type=Boolean  arith_type=Boolean  n_bits=5  bin_pt=0  preci_type=Single  exp_width=8  frac_width=24  explicit_period=on  period=4  equ=P=C  opselect=C  inp2=PCIN>>17  opr=+  inp1=P  carry=CIN  dbl_ovrd=off  block_type=constant
  FIFO : xbsIndex_r4/FIFO  mem_type=Block RAM  performance_options=Standard_FIFO  infoedit1=Available only for V4, V5, V6, V7, K7, A7 and Zynq devices.  embedded_registers=off  depth=64  percent_nbits=1  rst=off  en=off  use_dcount=off  use_percent_full_port=off  use_almost_empty=off  almost_empty_thresh=2  use_almost_full=off  almost_full_thresh=14  dbl_ovrd=off  explicit_period=off  block_type=fifo
  Reinterpret : xbsIndex_r4/Reinterpret  force_arith_type=on  arith_type=Unsigned  force_bin_pt=on  bin_pt=0  block_type=reinterpret
  Reinterpret1 : xbsIndex_r4/Reinterpret  force_arith_type=on  arith_type=Unsigned  force_bin_pt=on  bin_pt=0  block_type=reinterpret
  Reinterpret2 : xbsIndex_r4/Reinterpret  force_arith_type=on  arith_type="Signed  (2's comp)"  force_bin_pt=on  bin_pt=13  block_type=reinterpret
  Reinterpret3 : xbsIndex_r4/Reinterpret  force_arith_type=on  arith_type="Signed  (2's comp)"  force_bin_pt=on  bin_pt=13  block_type=reinterpret
  Up Sample : xbsIndex_r4/Up Sample  sample_ratio=4  copy_samples=on  en=off  latency=0  dbl_ovrd=off  block_type=usamp
  Up Sample1 : xbsIndex_r4/Up Sample  sample_ratio=4  copy_samples=on  en=off  latency=0  dbl_ovrd=off  block_type=usamp
  " I" : Outport
  " Q" : Outport  Port=2
  #Vout : Outport  Port=3
  wires:
  Constant3/1 -> FIFO/3
  Concat/1 -> FIFO/1
  I/1 -> Reinterpret/1
  14MSB/1 -> Reinterpret2/1
  Q/1 -> Reinterpret1/1
  14LSB/1 -> Reinterpret3/1
  Vin/1 -> FIFO/2
  FIFO/2 -> #Vout/1
  Reinterpret/1 -> Concat/1
  Reinterpret1/1 -> Concat/2
  Reinterpret2/1 -> Up Sample/1
  Reinterpret3/1 -> Up Sample1/1
  Up Sample/1 -> " I"/1
  Up Sample1/1 -> " Q"/1
  FIFO/1 -> 14LSB/1, 14MSB/1

== system wlan_phy_rx_pmd/Chan Est & EQ/EQ & Pilot Processing/Phase Err Calc/Accum1 ==
  note: Pilot tones must be spaced by more subcarriers
      | than latency of this addr. With natural order FFT
      | outputs worst case is 7->21 = 14 cycles
  En : Inport
  D : Inport  Port=2
  Rst : Inport  Port=3
  AddSub : xbsIndex_r4/AddSub  mode=Addition  use_carryin=off  use_carryout=off  en=on  latency=4  precision=Full  arith_type=Unsigned  n_bits=16  bin_pt=14  quantization=Truncate  overflow=Wrap  dbl_ovrd=off  use_behavioral_HDL=off  hw_selection=DSP48  pipelined=off  block_type=addsub
  Delay5 : xbsIndex_r4/Delay  rst=off  infoeditControl=Selection of Reset will increase slice count due to use of real FFs and instead of SRLs  en=off  latency=4  dbl_ovrd=off  reg_retiming=off  block_type=delay
  Register : xbsIndex_r4/Register  init=0  rst=on  en=on  dbl_ovrd=off  block_type=register
  Sum : Outport
  wires:
  Delay5/1 -> Register/3
  AddSub/1 -> Register/1, Sum/1
  En/1 -> Delay5/1, AddSub/3
  Rst/1 -> Register/2
  D/1 -> AddSub/1
  Register/1 -> AddSub/2

== system wlan_phy_rx_pmd/Chan Est & EQ/EQ & Pilot Processing/Phase Err Calc/Accum2 ==
  note: Pilot tones must be spaced by more subcarriers
      | than latency of this addr. With natural order FFT
      | outputs worst case is 7->21 = 14 cycles
  En : Inport
  D : Inport  Port=2
  Rst : Inport  Port=3
  AddSub : xbsIndex_r4/AddSub  mode=Addition  use_carryin=off  use_carryout=off  en=on  latency=4  precision=Full  arith_type=Unsigned  n_bits=16  bin_pt=14  quantization=Truncate  overflow=Wrap  dbl_ovrd=off  use_behavioral_HDL=off  hw_selection=DSP48  pipelined=off  block_type=addsub
  Delay5 : xbsIndex_r4/Delay  rst=off  infoeditControl=Selection of Reset will increase slice count due to use of real FFs and instead of SRLs  en=off  latency=4  dbl_ovrd=off  reg_retiming=off  block_type=delay
  Register : xbsIndex_r4/Register  init=0  rst=on  en=on  dbl_ovrd=off  block_type=register
  Sum : Outport
  wires:
  Register/1 -> AddSub/2
  D/1 -> AddSub/1
  Rst/1 -> Register/2
  En/1 -> AddSub/3, Delay5/1
  AddSub/1 -> Sum/1, Register/1
  Delay5/1 -> Register/3

== system wlan_phy_rx_pmd/Chan Est & EQ/EQ & Pilot Processing/Phase Err Calc/Count ==
  note: Latency to match phase accum latency
  En : Inport
  Rst : Inport  Port=2
  Constant3 : xbsIndex_r4/Constant  const=4  gui_display_data_type=Fixed-point  arith_type=Unsigned  n_bits=6  bin_pt=0  preci_type=Single  exp_width=8  frac_width=24  explicit_period=off  period=8  equ=P=C  opselect=C  inp2=PCIN>>17  opr=+  inp1=P  carry=CIN  dbl_ovrd=off  block_type=constant
  Counter : xbsIndex_r4/Counter  cnt_type=Free Running  cnt_to=Inf  operation=Up  start_count=0  cnt_by_val=1  arith_type=Unsigned  n_bits=3  bin_pt=0  load_pin=off  rst=on  en=on  explicit_period=off  period=1  dbl_ovrd=off  use_behavioral_HDL=off  implementation=Fabric  block_type=counter
  Delay : xbsIndex_r4/Delay  rst=off  infoeditControl=Selection of Reset will increase slice count due to use of real FFs and instead of SRLs  en=off  latency=1  dbl_ovrd=off  reg_retiming=off  block_type=delay
  Delay1 : xbsIndex_r4/Delay  rst=off  infoeditControl=Selection of Reset will increase slice count due to use of real FFs and instead of SRLs  en=off  latency=4  dbl_ovrd=off  reg_retiming=off  block_type=delay
  Logical : xbsIndex_r4/Logical  logical_function=AND  inputs=2  en=off  latency=0  precision=Full  arith_type=Unsigned  n_bits=16  bin_pt=0  align_bp=on  dbl_ovrd=off  block_type=logical
  Relational7 : xbsIndex_r4/Relational  mode=a=b  en=off  latency=0  dbl_ovrd=off  block_type=relational
      settings: xl_area=[2 0 0 3 0 0 0]  block_version=VER_STRING_GOES_HERE
  Last : Outport
  wires:
  Counter/1 -> Relational7/2
  Constant3/1 -> Relational7/1
  Relational7/1 -> Logical/1
  En/1 -> Counter/2, Delay/1
  Rst/1 -> Counter/1
  Logical/1 -> Delay1/1
  Delay/1 -> Logical/2
  Delay1/1 -> Last/1

== system wlan_phy_rx_pmd/Chan Est & EQ/EQ & Pilot Processing/Phase Err Calc/Descramble ==
  Negate : Inport
  x_I : Inport  Port=2
  x_Q : Inport  Port=3
  Sym Ind : Inport  Port=4
  Constant2 : xbsIndex_r4/Constant  const=3  gui_display_data_type=Fixed-point  arith_type=Unsigned  n_bits=2  bin_pt=0  preci_type=Single  exp_width=8  frac_width=24  explicit_period=off  period=8  equ=P=C  opselect=C  inp2=PCIN>>17  opr=+  inp1=P  carry=CIN  dbl_ovrd=off  block_type=constant
  Delay : xbsIndex_r4/Delay  rst=off  infoeditControl=Selection of Reset will increase slice count due to use of real FFs and instead of SRLs  en=off  latency=1  dbl_ovrd=off  reg_retiming=off  block_type=delay
  Delay1 : xbsIndex_r4/Delay  rst=off  infoeditControl=Selection of Reset will increase slice count due to use of real FFs and instead of SRLs  en=off  latency=1  dbl_ovrd=off  reg_retiming=off  block_type=delay
  Delay2 : xbsIndex_r4/Delay  rst=off  infoeditControl=Selection of Reset will increase slice count due to use of real FFs and instead of SRLs  en=off  latency=1  dbl_ovrd=off  reg_retiming=off  block_type=delay
  Logical : xbsIndex_r4/Logical  logical_function=XOR  inputs=2  en=off  latency=0  precision=Full  arith_type=Unsigned  n_bits=8  bin_pt=2  align_bp=on  dbl_ovrd=off  block_type=logical
      settings: xl_area=[0, 0, 0, 0, 0, 0, 0]  block_version=VER_STRING_GOES_HERE
  Mux1 : xbsIndex_r4/Mux  inputs=2  en=off  latency=0  precision=Full  arith_type="Signed  (2's comp)"  n_bits=8  bin_pt=2  quantization=Truncate  overflow=Wrap  dbl_ovrd=off  block_type=mux
      settings: xl_area=[16 0 0 32 0 0 0]  block_version=VER_STRING_GOES_HERE
  Mux2 : xbsIndex_r4/Mux  inputs=2  en=off  latency=0  precision=Full  arith_type="Signed  (2's comp)"  n_bits=8  bin_pt=2  quantization=Truncate  overflow=Wrap  dbl_ovrd=off  block_type=mux
      settings: xl_area=[16 0 0 32 0 0 0]  block_version=VER_STRING_GOES_HERE
  Negate1 : xbsIndex_r4/Negate  precision=Full  arith_type="Signed  (2's comp)"  n_bits=17  bin_pt=15  quantization="Round  (unbiased: +/- Inf)"  overflow=Saturate  en=off  latency=1  dbl_ovrd=off  block_type=negate
      settings: block_version=VER_STRING_GOES_HERE
  Negate2 : xbsIndex_r4/Negate  precision=Full  arith_type="Signed  (2's comp)"  n_bits=17  bin_pt=15  quantization="Round  (unbiased: +/- Inf)"  overflow=Saturate  en=off  latency=1  dbl_ovrd=off  block_type=negate
      settings: block_version=VER_STRING_GOES_HERE
  Relational2 : xbsIndex_r4/Relational  mode=a<b  en=off  latency=0  dbl_ovrd=off  block_type=relational
  Scrambling LFSR : Subsystem
  edge : Subsystem
  " x_I" : Outport
  " x_Q" : Outport  Port=2
  wires:
  Constant2/1 -> Relational2/2
  edge/1(Q) -> Scrambling LFSR/1(en)
  Relational2/1 -> Scrambling LFSR/2(rst)
  Scrambling LFSR/1(Q) -> Logical/2
  Logical/1 -> Delay2/1
  Negate1/1 -> Mux1/3
  x_I/1 -> Negate1/1, Delay/1
  Sym Ind/1 -> edge/1(D), Relational2/1
  Negate/1 -> Logical/1
  Mux1/1 -> " x_I"/1
  Negate2/1 -> Mux2/3
  x_Q/1 -> Negate2/1, Delay1/1
  Mux2/1 -> " x_Q"/1
  Delay/1 -> Mux1/2
  Delay1/1 -> Mux2/2
  Delay2/1 -> Mux1/1, Mux2/1
